simulink library "Garbage"  (R2023a)
settings: Solver=ode45  StartTime=0.0  StopTime=10.0  RelTol=1e-3  EnableMultiTasking=on
undefined symbols (not defined in the file or in its folder): c, ending_column_index, ending_row_index, framebuffer_16x1, framebuffer_2x2, framebuffer_4x4, framebuffer_8x8, HEIGHT, hot, POS_X, POS_Y, starting_column_index, starting_row_index, WIDTH
libraries: Memory_Blocks_Lib x459, simulink x235, simulink_extras x132, dspmtrx3 x116, Bit_Multiplexers_Lib x67, Bit_Demultiplexers_Lib x49, Byte_Multiplexers_Lib x40, Miscellaneous x38, Computer_Library x17, Decoders_Lib x14, commutil2 x12, Arithmetic_Operations_Lib x8
(part 11 of 26)

== system Garbage/NOT_USED_Byte_Demultiplexer_1_to_4 ==
  Addr_1(1b) : Inport
  Addr_0(1b) : Inport  Port=2
  Data(1B) : Inport  Port=3
  1_to_4_Bit_Demultiplexer(Bit_0) : Bit_Demultiplexers_Lib/Bit_Demultiplexers_Lib/Bit_Demultiplexer_1_to_4
  1_to_4_Bit_Demultiplexer(Bit_1) : Bit_Demultiplexers_Lib/Bit_Demultiplexers_Lib/Bit_Demultiplexer_1_to_4
  1_to_4_Bit_Demultiplexer(Bit_2) : Bit_Demultiplexers_Lib/Bit_Demultiplexers_Lib/Bit_Demultiplexer_1_to_4
  1_to_4_Bit_Demultiplexer(Bit_3) : Bit_Demultiplexers_Lib/Bit_Demultiplexers_Lib/Bit_Demultiplexer_1_to_4
  1_to_4_Bit_Demultiplexer(Bit_4) : Bit_Demultiplexers_Lib/Bit_Demultiplexers_Lib/Bit_Demultiplexer_1_to_4
  1_to_4_Bit_Demultiplexer(Bit_5) : Bit_Demultiplexers_Lib/Bit_Demultiplexers_Lib/Bit_Demultiplexer_1_to_4
  1_to_4_Bit_Demultiplexer(Bit_6) : Bit_Demultiplexers_Lib/Bit_Demultiplexers_Lib/Bit_Demultiplexer_1_to_4
  1_to_4_Bit_Demultiplexer(Bit_7) : Bit_Demultiplexers_Lib/Bit_Demultiplexers_Lib/Bit_Demultiplexer_1_to_4
  Bit to Integer Converter{,1..3} (4 alike) : commutil2/Bit to Integer Converter  nbits=8  bitOrder=MSB first  signedOutputValues=Unsigned  outDtype=uint8  outDtypeSigned=Inherit via internal rule
  Byte_{0..3} (4 alike) : Mux  Inputs=8
  Demux1 : Demux  Outputs=8
  Integer to Bit Converter7 : commutil2/Integer to Bit Converter  nbits=8  signedInputValues=Unsigned  bitOrder=MSB first  outDtype=boolean
  Data_3(1B) : Outport
  Data_2(1B) : Outport  Port=2
  Data_1(1B) : Outport  Port=3
  Data_0(1B) : Outport  Port=4
  wires:
  Bit to Integer Converter/1 -> Data_3(1B)/1
  Demux1/4 -> 1_to_4_Bit_Demultiplexer(Bit_4)/3
  Demux1/5 -> 1_to_4_Bit_Demultiplexer(Bit_3)/3
  Demux1/6 -> 1_to_4_Bit_Demultiplexer(Bit_2)/3
  Demux1/7 -> 1_to_4_Bit_Demultiplexer(Bit_1)/3
  Demux1/8 -> 1_to_4_Bit_Demultiplexer(Bit_0)/3
  Demux1/3 -> 1_to_4_Bit_Demultiplexer(Bit_5)/3
  Demux1/2 -> 1_to_4_Bit_Demultiplexer(Bit_6)/3
  Demux1/1 -> 1_to_4_Bit_Demultiplexer(Bit_7)/3
  Byte_3/1 -> Bit to Integer Converter/1
  Byte_2/1 -> Bit to Integer Converter1/1
  Byte_1/1 -> Bit to Integer Converter2/1
  Byte_0/1 -> Bit to Integer Converter3/1
  1_to_4_Bit_Demultiplexer(Bit_0)/4 -> Byte_0/8
  1_to_4_Bit_Demultiplexer(Bit_0)/3 -> Byte_1/8
  1_to_4_Bit_Demultiplexer(Bit_0)/2 -> Byte_2/8
  1_to_4_Bit_Demultiplexer(Bit_0)/1 -> Byte_3/8
  1_to_4_Bit_Demultiplexer(Bit_1)/4 -> Byte_0/7
  1_to_4_Bit_Demultiplexer(Bit_1)/3 -> Byte_1/7
  1_to_4_Bit_Demultiplexer(Bit_1)/2 -> Byte_2/7
  1_to_4_Bit_Demultiplexer(Bit_1)/1 -> Byte_3/7
  1_to_4_Bit_Demultiplexer(Bit_2)/4 -> Byte_0/6
  1_to_4_Bit_Demultiplexer(Bit_2)/3 -> Byte_1/6
  1_to_4_Bit_Demultiplexer(Bit_2)/2 -> Byte_2/6
  1_to_4_Bit_Demultiplexer(Bit_2)/1 -> Byte_3/6
  1_to_4_Bit_Demultiplexer(Bit_3)/4 -> Byte_0/5
  1_to_4_Bit_Demultiplexer(Bit_3)/3 -> Byte_1/5
  1_to_4_Bit_Demultiplexer(Bit_3)/2 -> Byte_2/5
  1_to_4_Bit_Demultiplexer(Bit_3)/1 -> Byte_3/5
  1_to_4_Bit_Demultiplexer(Bit_4)/4 -> Byte_0/4
  1_to_4_Bit_Demultiplexer(Bit_4)/3 -> Byte_1/4
  1_to_4_Bit_Demultiplexer(Bit_4)/2 -> Byte_2/4
  1_to_4_Bit_Demultiplexer(Bit_4)/1 -> Byte_3/4
  1_to_4_Bit_Demultiplexer(Bit_5)/4 -> Byte_0/3
  1_to_4_Bit_Demultiplexer(Bit_5)/3 -> Byte_1/3
  1_to_4_Bit_Demultiplexer(Bit_5)/2 -> Byte_2/3
  1_to_4_Bit_Demultiplexer(Bit_5)/1 -> Byte_3/3
  1_to_4_Bit_Demultiplexer(Bit_6)/4 -> Byte_0/2
  1_to_4_Bit_Demultiplexer(Bit_6)/3 -> Byte_1/2
  1_to_4_Bit_Demultiplexer(Bit_6)/2 -> Byte_2/2
  1_to_4_Bit_Demultiplexer(Bit_6)/1 -> Byte_3/2
  1_to_4_Bit_Demultiplexer(Bit_7)/4 -> Byte_0/1
  1_to_4_Bit_Demultiplexer(Bit_7)/3 -> Byte_1/1
  1_to_4_Bit_Demultiplexer(Bit_7)/2 -> Byte_2/1
  1_to_4_Bit_Demultiplexer(Bit_7)/1 -> Byte_3/1
  Integer to Bit Converter7/1 -> Demux1/1
  Data(1B)/1 -> Integer to Bit Converter7/1
  Addr_0(1b)/1 -> 1_to_4_Bit_Demultiplexer(Bit_7)/2, 1_to_4_Bit_Demultiplexer(Bit_3)/2, 1_to_4_Bit_Demultiplexer(Bit_2)/2, 1_to_4_Bit_Demultiplexer(Bit_1)/2, 1_to_4_Bit_Demultiplexer(Bit_0)/2, 1_to_4_Bit_Demultiplexer(Bit_4)/2, 1_to_4_Bit_Demultiplexer(Bit_5)/2, 1_to_4_Bit_Demultiplexer(Bit_6)/2
  Addr_1(1b)/1 -> 1_to_4_Bit_Demultiplexer(Bit_7)/1, 1_to_4_Bit_Demultiplexer(Bit_0)/1, 1_to_4_Bit_Demultiplexer(Bit_1)/1, 1_to_4_Bit_Demultiplexer(Bit_2)/1, 1_to_4_Bit_Demultiplexer(Bit_3)/1, 1_to_4_Bit_Demultiplexer(Bit_4)/1, 1_to_4_Bit_Demultiplexer(Bit_5)/1, 1_to_4_Bit_Demultiplexer(Bit_6)/1
  Bit to Integer Converter1/1 -> Data_2(1B)/1
  Bit to Integer Converter2/1 -> Data_1(1B)/1
  Bit to Integer Converter3/1 -> Data_0(1B)/1

== system Garbage/NOT_USED_Byte_Demultiplexer_1_to_8 ==
  Addr_2(1b) : Inport
  Addr_1(1b) : Inport  Port=2
  Addr_0(1b) : Inport  Port=3
  Data(1B) : Inport  Port=4
  1_to_8_Bit_Demultiplexer(Bit_0) : Bit_Demultiplexers_Lib/Bit_Demultiplexers_Lib/Bit_Demultiplexer_1_to_8
  1_to_8_Bit_Demultiplexer(Bit_1) : Bit_Demultiplexers_Lib/Bit_Demultiplexers_Lib/Bit_Demultiplexer_1_to_8
  1_to_8_Bit_Demultiplexer(Bit_2) : Bit_Demultiplexers_Lib/Bit_Demultiplexers_Lib/Bit_Demultiplexer_1_to_8
  1_to_8_Bit_Demultiplexer(Bit_3) : Bit_Demultiplexers_Lib/Bit_Demultiplexers_Lib/Bit_Demultiplexer_1_to_8
  1_to_8_Bit_Demultiplexer(Bit_4) : Bit_Demultiplexers_Lib/Bit_Demultiplexers_Lib/Bit_Demultiplexer_1_to_8
  1_to_8_Bit_Demultiplexer(Bit_5) : Bit_Demultiplexers_Lib/Bit_Demultiplexers_Lib/Bit_Demultiplexer_1_to_8
  1_to_8_Bit_Demultiplexer(Bit_6) : Bit_Demultiplexers_Lib/Bit_Demultiplexers_Lib/Bit_Demultiplexer_1_to_8
  1_to_8_Bit_Demultiplexer(Bit_7) : Bit_Demultiplexers_Lib/Bit_Demultiplexers_Lib/Bit_Demultiplexer_1_to_8
  Bus Creator{1..7,15} (8 alike) : BusCreator  Inputs=8
  Demux1 : Demux  Outputs=8
  Data_7(1B) : Outport
  Data_6(1B) : Outport  Port=2
  Data_5(1B) : Outport  Port=3
  Data_4(1B) : Outport  Port=4
  Data_3(1B) : Outport  Port=5
  Data_2(1B) : Outport  Port=6
  Data_1(1B) : Outport  Port=7
  Data_0(1B) : Outport  Port=8
  wires:
  Demux1/4 -> 1_to_8_Bit_Demultiplexer(Bit_4)/4
  Demux1/5 -> 1_to_8_Bit_Demultiplexer(Bit_3)/4
  Demux1/6 -> 1_to_8_Bit_Demultiplexer(Bit_2)/4
  Demux1/7 -> 1_to_8_Bit_Demultiplexer(Bit_1)/4
  Demux1/8 -> 1_to_8_Bit_Demultiplexer(Bit_0)/4
  Demux1/3 -> 1_to_8_Bit_Demultiplexer(Bit_5)/4
  Demux1/2 -> 1_to_8_Bit_Demultiplexer(Bit_6)/4
  Demux1/1 -> 1_to_8_Bit_Demultiplexer(Bit_7)/4
  1_to_8_Bit_Demultiplexer(Bit_0)/8 -> Bus Creator7/8
  1_to_8_Bit_Demultiplexer(Bit_0)/7 -> Bus Creator6/8
  1_to_8_Bit_Demultiplexer(Bit_0)/6 -> Bus Creator5/8
  1_to_8_Bit_Demultiplexer(Bit_0)/5 -> Bus Creator4/8
  1_to_8_Bit_Demultiplexer(Bit_0)/4 -> Bus Creator3/8
  1_to_8_Bit_Demultiplexer(Bit_0)/3 -> Bus Creator2/8
  1_to_8_Bit_Demultiplexer(Bit_0)/2 -> Bus Creator1/8
  1_to_8_Bit_Demultiplexer(Bit_0)/1 -> Bus Creator15/8
  1_to_8_Bit_Demultiplexer(Bit_1)/8 -> Bus Creator7/7
  1_to_8_Bit_Demultiplexer(Bit_1)/7 -> Bus Creator6/7
  1_to_8_Bit_Demultiplexer(Bit_1)/6 -> Bus Creator5/7
  1_to_8_Bit_Demultiplexer(Bit_1)/5 -> Bus Creator4/7
  1_to_8_Bit_Demultiplexer(Bit_1)/4 -> Bus Creator3/7
  1_to_8_Bit_Demultiplexer(Bit_1)/3 -> Bus Creator2/7
  1_to_8_Bit_Demultiplexer(Bit_1)/2 -> Bus Creator1/7
  1_to_8_Bit_Demultiplexer(Bit_1)/1 -> Bus Creator15/7
  1_to_8_Bit_Demultiplexer(Bit_2)/8 -> Bus Creator7/6
  1_to_8_Bit_Demultiplexer(Bit_2)/7 -> Bus Creator6/6
  1_to_8_Bit_Demultiplexer(Bit_2)/6 -> Bus Creator5/6
  1_to_8_Bit_Demultiplexer(Bit_2)/5 -> Bus Creator4/6
  1_to_8_Bit_Demultiplexer(Bit_2)/4 -> Bus Creator3/6
  1_to_8_Bit_Demultiplexer(Bit_2)/3 -> Bus Creator2/6
  1_to_8_Bit_Demultiplexer(Bit_2)/2 -> Bus Creator1/6
  1_to_8_Bit_Demultiplexer(Bit_2)/1 -> Bus Creator15/6
  1_to_8_Bit_Demultiplexer(Bit_3)/8 -> Bus Creator7/5
  1_to_8_Bit_Demultiplexer(Bit_3)/7 -> Bus Creator6/5
  1_to_8_Bit_Demultiplexer(Bit_3)/6 -> Bus Creator5/5
  1_to_8_Bit_Demultiplexer(Bit_3)/5 -> Bus Creator4/5
  1_to_8_Bit_Demultiplexer(Bit_3)/4 -> Bus Creator3/5
  1_to_8_Bit_Demultiplexer(Bit_3)/3 -> Bus Creator2/5
  1_to_8_Bit_Demultiplexer(Bit_3)/2 -> Bus Creator1/5
  1_to_8_Bit_Demultiplexer(Bit_3)/1 -> Bus Creator15/5
  1_to_8_Bit_Demultiplexer(Bit_4)/8 -> Bus Creator7/4
  1_to_8_Bit_Demultiplexer(Bit_4)/7 -> Bus Creator6/4
  1_to_8_Bit_Demultiplexer(Bit_4)/6 -> Bus Creator5/4
  1_to_8_Bit_Demultiplexer(Bit_4)/5 -> Bus Creator4/4
  1_to_8_Bit_Demultiplexer(Bit_4)/4 -> Bus Creator3/4
  1_to_8_Bit_Demultiplexer(Bit_4)/3 -> Bus Creator2/4
  1_to_8_Bit_Demultiplexer(Bit_4)/2 -> Bus Creator1/4
  1_to_8_Bit_Demultiplexer(Bit_4)/1 -> Bus Creator15/4
  1_to_8_Bit_Demultiplexer(Bit_5)/8 -> Bus Creator7/3
  1_to_8_Bit_Demultiplexer(Bit_5)/7 -> Bus Creator6/3
  1_to_8_Bit_Demultiplexer(Bit_5)/6 -> Bus Creator5/3
  1_to_8_Bit_Demultiplexer(Bit_5)/5 -> Bus Creator4/3
  1_to_8_Bit_Demultiplexer(Bit_5)/4 -> Bus Creator3/3
  1_to_8_Bit_Demultiplexer(Bit_5)/3 -> Bus Creator2/3
  1_to_8_Bit_Demultiplexer(Bit_5)/2 -> Bus Creator1/3
  1_to_8_Bit_Demultiplexer(Bit_5)/1 -> Bus Creator15/3
  1_to_8_Bit_Demultiplexer(Bit_6)/8 -> Bus Creator7/2
  1_to_8_Bit_Demultiplexer(Bit_6)/7 -> Bus Creator6/2
  1_to_8_Bit_Demultiplexer(Bit_6)/6 -> Bus Creator5/2
  1_to_8_Bit_Demultiplexer(Bit_6)/5 -> Bus Creator4/2
  1_to_8_Bit_Demultiplexer(Bit_6)/4 -> Bus Creator3/2
  1_to_8_Bit_Demultiplexer(Bit_6)/3 -> Bus Creator2/2
  1_to_8_Bit_Demultiplexer(Bit_6)/2 -> Bus Creator1/2
  1_to_8_Bit_Demultiplexer(Bit_6)/1 -> Bus Creator15/2
  1_to_8_Bit_Demultiplexer(Bit_7)/8 -> Bus Creator7/1
  1_to_8_Bit_Demultiplexer(Bit_7)/7 -> Bus Creator6/1
  1_to_8_Bit_Demultiplexer(Bit_7)/6 -> Bus Creator5/1
  1_to_8_Bit_Demultiplexer(Bit_7)/5 -> Bus Creator4/1
  1_to_8_Bit_Demultiplexer(Bit_7)/4 -> Bus Creator3/1
  1_to_8_Bit_Demultiplexer(Bit_7)/3 -> Bus Creator2/1
  1_to_8_Bit_Demultiplexer(Bit_7)/2 -> Bus Creator1/1
  1_to_8_Bit_Demultiplexer(Bit_7)/1 -> Bus Creator15/1
  Data(1B)/1 -> Demux1/1
  Addr_0(1b)/1 -> 1_to_8_Bit_Demultiplexer(Bit_7)/3, 1_to_8_Bit_Demultiplexer(Bit_6)/3, 1_to_8_Bit_Demultiplexer(Bit_5)/3, 1_to_8_Bit_Demultiplexer(Bit_0)/3, 1_to_8_Bit_Demultiplexer(Bit_1)/3, 1_to_8_Bit_Demultiplexer(Bit_2)/3, 1_to_8_Bit_Demultiplexer(Bit_3)/3, 1_to_8_Bit_Demultiplexer(Bit_4)/3
  Addr_1(1b)/1 -> 1_to_8_Bit_Demultiplexer(Bit_7)/2, 1_to_8_Bit_Demultiplexer(Bit_0)/2, 1_to_8_Bit_Demultiplexer(Bit_1)/2, 1_to_8_Bit_Demultiplexer(Bit_2)/2, 1_to_8_Bit_Demultiplexer(Bit_3)/2, 1_to_8_Bit_Demultiplexer(Bit_4)/2, 1_to_8_Bit_Demultiplexer(Bit_5)/2, 1_to_8_Bit_Demultiplexer(Bit_6)/2
  Addr_2(1b)/1 -> 1_to_8_Bit_Demultiplexer(Bit_7)/1, 1_to_8_Bit_Demultiplexer(Bit_0)/1, 1_to_8_Bit_Demultiplexer(Bit_1)/1, 1_to_8_Bit_Demultiplexer(Bit_2)/1, 1_to_8_Bit_Demultiplexer(Bit_3)/1, 1_to_8_Bit_Demultiplexer(Bit_4)/1, 1_to_8_Bit_Demultiplexer(Bit_5)/1, 1_to_8_Bit_Demultiplexer(Bit_6)/1
  Bus Creator7/1 -> Data_0(1B)/1
  Bus Creator6/1 -> Data_1(1B)/1
  Bus Creator5/1 -> Data_2(1B)/1
  Bus Creator4/1 -> Data_3(1B)/1
  Bus Creator3/1 -> Data_4(1B)/1
  Bus Creator2/1 -> Data_5(1B)/1
  Bus Creator1/1 -> Data_6(1B)/1
  Bus Creator15/1 -> Data_7(1B)/1

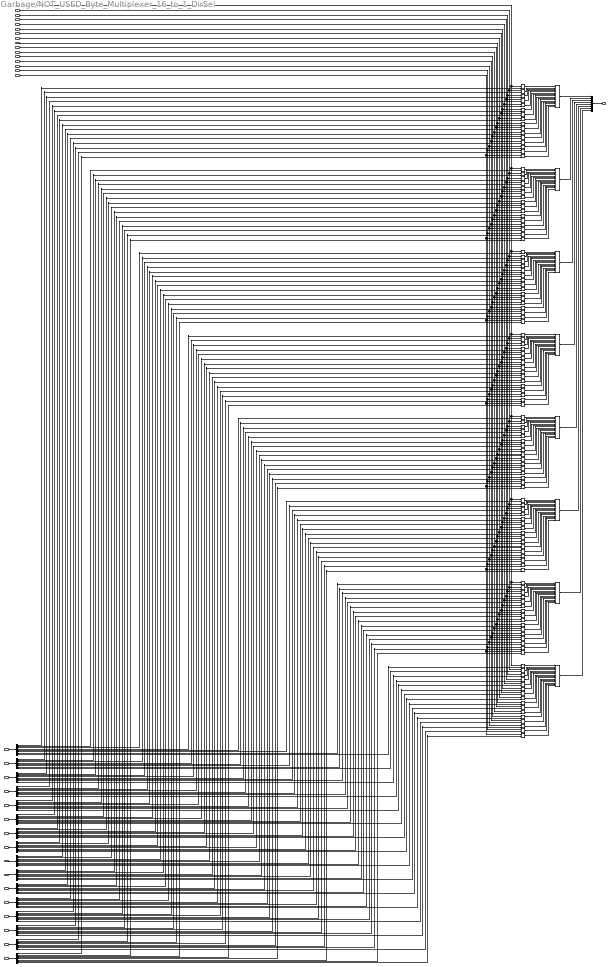
== system Garbage/NOT_USED_Byte_Multiplexer_16_to_1_DirSel ==
  note: Bit_Multiplexer_16_to_1_DirSel(Bit_07)
  note: Bit_Multiplexer_16_to_1_DirSel(Bit_06)
  note: Bit_Multiplexer_16_to_1_DirSel(Bit_05)
  note: Bit_Multiplexer_16_to_1_DirSel(Bit_04)
  note: Bit_Multiplexer_16_to_1_DirSel(Bit_03)
  note: Bit_Multiplexer_16_to_1_DirSel(Bit_02)
  note: Bit_Multiplexer_16_to_1_DirSel(Bit_01)
  note: Bit_Multiplexer_16_to_1_DirSel(Bit_00)
  Select_15(1b) : Inport
  Select_14(1b) : Inport  Port=2
  Select_13(1b) : Inport  Port=3
  Select_12(1b) : Inport  Port=4
  Select_11(1b) : Inport  Port=5
  Select_10(1b) : Inport  Port=6
  Select_09(1b) : Inport  Port=7
  Select_08(1b) : Inport  Port=8
  Select_07(1b) : Inport  Port=9
  Select_06(1b) : Inport  Port=10
  Select_05(1b) : Inport  Port=11
  Select_04(1b) : Inport  Port=12
  Select_03(1b) : Inport  Port=13
  Select_02(1b) : Inport  Port=14
  Select_01(1b) : Inport  Port=15
  Select_00(1b) : Inport  Port=16
  Data_15(1B) : Inport  Port=17
  Data_14(1B) : Inport  Port=18
  Data_13(1B) : Inport  Port=19
  Data_12(1B) : Inport  Port=20
  Data_11(1B) : Inport  Port=21
  Data_10(1B) : Inport  Port=22
  Data_09(1B) : Inport  Port=23
  Data_08(1B) : Inport  Port=24
  Data_07(1B) : Inport  Port=25
  Data_06(1B) : Inport  Port=26
  Data_05(1B) : Inport  Port=27
  Data_04(1B) : Inport  Port=28
  Data_03(1B) : Inport  Port=29
  Data_02(1B) : Inport  Port=30
  Data_01(1B) : Inport  Port=31
  Data_00(1B) : Inport  Port=32
  Bus Creator : BusCreator  Inputs=8
  Bus Selector{1..16} (16 alike) : BusSelector  OutputSignals=signal1,signal2,signal3,signal4,signal5,signal6,signal7,signal8
  Logical Operator{137..151,153..168,170..185,187..202,204..219,221..236,238..253,255..270,272} (128 alike) : Logic  IconShape=distinctive  AllPortsSameDT=off  OutDataTypeStr=boolean
  Logical Operator{152,169,186,203,220,237,254,271} (8 alike) : Logic  Operator=OR  Inputs=16  IconShape=distinctive  OutDataTypeStr=boolean
  Data(1B) : Outport
  wires:
  Data_15(1B)/1 -> Bus Selector15/1
  Data_14(1B)/1 -> Bus Selector1/1
  Data_13(1B)/1 -> Bus Selector2/1
  Data_12(1B)/1 -> Bus Selector3/1
  Data_11(1B)/1 -> Bus Selector4/1
  Data_10(1B)/1 -> Bus Selector5/1
  Data_09(1B)/1 -> Bus Selector6/1
  Data_08(1B)/1 -> Bus Selector7/1
  Data_07(1B)/1 -> Bus Selector8/1
  Data_06(1B)/1 -> Bus Selector9/1
  Data_05(1B)/1 -> Bus Selector10/1
  Data_04(1B)/1 -> Bus Selector11/1
  Data_03(1B)/1 -> Bus Selector12/1
  Data_02(1B)/1 -> Bus Selector13/1
  Data_01(1B)/1 -> Bus Selector14/1
  Data_00(1B)/1 -> Bus Selector16/1
  Bus Creator/1 -> Data(1B)/1
  Bus Selector16/8 -> Logical Operator264/2  "<signal8>"
  Bus Selector14/8 -> Logical Operator263/2  "<signal8>"
  Bus Selector13/8 -> Logical Operator262/2  "<signal8>"
  Bus Selector12/8 -> Logical Operator261/2  "<signal8>"
  Bus Selector11/8 -> Logical Operator258/2  "<signal8>"
  Bus Selector10/8 -> Logical Operator257/2  "<signal8>"
  Bus Selector9/8 -> Logical Operator256/2  "<signal8>"
  Bus Selector8/8 -> Logical Operator272/2  "<signal8>"
  Bus Selector15/8 -> Logical Operator259/2  "<signal8>"
  Bus Selector1/8 -> Logical Operator260/2  "<signal8>"
  Bus Selector2/8 -> Logical Operator265/2  "<signal8>"
  Bus Selector3/8 -> Logical Operator266/2  "<signal8>"
  Bus Selector4/8 -> Logical Operator267/2  "<signal8>"
  Bus Selector5/8 -> Logical Operator268/2  "<signal8>"
  Bus Selector6/8 -> Logical Operator269/2  "<signal8>"
  Bus Selector7/8 -> Logical Operator270/2  "<signal8>"
  Bus Selector16/7 -> Logical Operator247/2  "<signal7>"
  Bus Selector14/7 -> Logical Operator246/2  "<signal7>"
  Bus Selector13/7 -> Logical Operator245/2  "<signal7>"
  Bus Selector12/7 -> Logical Operator244/2  "<signal7>"
  Bus Selector11/7 -> Logical Operator241/2  "<signal7>"
  Bus Selector10/7 -> Logical Operator240/2  "<signal7>"
  Bus Selector9/7 -> Logical Operator239/2  "<signal7>"
  Bus Selector8/7 -> Logical Operator255/2  "<signal7>"
  Bus Selector15/7 -> Logical Operator242/2  "<signal7>"
  Bus Selector1/7 -> Logical Operator243/2  "<signal7>"
  Bus Selector2/7 -> Logical Operator248/2  "<signal7>"
  Bus Selector3/7 -> Logical Operator249/2  "<signal7>"
  Bus Selector4/7 -> Logical Operator250/2  "<signal7>"
  Bus Selector5/7 -> Logical Operator251/2  "<signal7>"
  Bus Selector6/7 -> Logical Operator252/2  "<signal7>"
  Bus Selector7/7 -> Logical Operator253/2  "<signal7>"
  Bus Selector16/6 -> Logical Operator230/2  "<signal6>"
  Bus Selector14/6 -> Logical Operator229/2  "<signal6>"
  Bus Selector13/6 -> Logical Operator228/2  "<signal6>"
  Bus Selector12/6 -> Logical Operator227/2  "<signal6>"
  Bus Selector11/6 -> Logical Operator224/2  "<signal6>"
  Bus Selector10/6 -> Logical Operator223/2  "<signal6>"
  Bus Selector9/6 -> Logical Operator222/2  "<signal6>"
  Bus Selector8/6 -> Logical Operator238/2  "<signal6>"
  Bus Selector15/6 -> Logical Operator225/2  "<signal6>"
  Bus Selector1/6 -> Logical Operator226/2  "<signal6>"
  Bus Selector2/6 -> Logical Operator231/2  "<signal6>"
  Bus Selector3/6 -> Logical Operator232/2  "<signal6>"
  Bus Selector4/6 -> Logical Operator233/2  "<signal6>"
  Bus Selector5/6 -> Logical Operator234/2  "<signal6>"
  Bus Selector6/6 -> Logical Operator235/2  "<signal6>"
  Bus Selector7/6 -> Logical Operator236/2  "<signal6>"
  Bus Selector16/5 -> Logical Operator213/2  "<signal5>"
  Bus Selector14/5 -> Logical Operator212/2  "<signal5>"
  Bus Selector13/5 -> Logical Operator211/2  "<signal5>"
  Bus Selector12/5 -> Logical Operator210/2  "<signal5>"
  Bus Selector11/5 -> Logical Operator207/2  "<signal5>"
  Bus Selector10/5 -> Logical Operator206/2  "<signal5>"
  Bus Selector9/5 -> Logical Operator205/2  "<signal5>"
  Bus Selector8/5 -> Logical Operator221/2  "<signal5>"
  Bus Selector15/5 -> Logical Operator208/2  "<signal5>"
  Bus Selector1/5 -> Logical Operator209/2  "<signal5>"
  Bus Selector2/5 -> Logical Operator214/2  "<signal5>"
  Bus Selector3/5 -> Logical Operator215/2  "<signal5>"
  Bus Selector4/5 -> Logical Operator216/2  "<signal5>"
  Bus Selector5/5 -> Logical Operator217/2  "<signal5>"
  Bus Selector6/5 -> Logical Operator218/2  "<signal5>"
  Bus Selector7/5 -> Logical Operator219/2  "<signal5>"
  Bus Selector16/4 -> Logical Operator196/2  "<signal4>"
  Bus Selector14/4 -> Logical Operator195/2  "<signal4>"
  Bus Selector13/4 -> Logical Operator194/2  "<signal4>"
  Bus Selector12/4 -> Logical Operator193/2  "<signal4>"
  Bus Selector11/4 -> Logical Operator190/2  "<signal4>"
  Bus Selector10/4 -> Logical Operator189/2  "<signal4>"
  Bus Selector9/4 -> Logical Operator188/2  "<signal4>"
  Bus Selector8/4 -> Logical Operator204/2  "<signal4>"
  Bus Selector15/4 -> Logical Operator191/2  "<signal4>"
  Bus Selector1/4 -> Logical Operator192/2  "<signal4>"
  Bus Selector2/4 -> Logical Operator197/2  "<signal4>"
  Bus Selector3/4 -> Logical Operator198/2  "<signal4>"
  Bus Selector4/4 -> Logical Operator199/2  "<signal4>"
  Bus Selector5/4 -> Logical Operator200/2  "<signal4>"
  Bus Selector6/4 -> Logical Operator201/2  "<signal4>"
  Bus Selector7/4 -> Logical Operator202/2  "<signal4>"
  Bus Selector16/3 -> Logical Operator179/2  "<signal3>"
  Bus Selector14/3 -> Logical Operator178/2  "<signal3>"
  Bus Selector13/3 -> Logical Operator177/2  "<signal3>"
  Bus Selector12/3 -> Logical Operator176/2  "<signal3>"
  Bus Selector11/3 -> Logical Operator173/2  "<signal3>"
  Bus Selector10/3 -> Logical Operator172/2  "<signal3>"
  Bus Selector9/3 -> Logical Operator171/2  "<signal3>"
  Bus Selector8/3 -> Logical Operator187/2  "<signal3>"
  Bus Selector15/3 -> Logical Operator174/2  "<signal3>"
  Bus Selector1/3 -> Logical Operator175/2  "<signal3>"
  Bus Selector2/3 -> Logical Operator180/2  "<signal3>"
  Bus Selector3/3 -> Logical Operator181/2  "<signal3>"
  Bus Selector4/3 -> Logical Operator182/2  "<signal3>"
  Bus Selector5/3 -> Logical Operator183/2  "<signal3>"
  Bus Selector6/3 -> Logical Operator184/2  "<signal3>"
  Bus Selector7/3 -> Logical Operator185/2  "<signal3>"
  Bus Selector16/2 -> Logical Operator162/2  "<signal2>"
  Bus Selector14/2 -> Logical Operator161/2  "<signal2>"
  Bus Selector13/2 -> Logical Operator160/2  "<signal2>"
  Bus Selector12/2 -> Logical Operator159/2  "<signal2>"
  Bus Selector11/2 -> Logical Operator156/2  "<signal2>"
  Bus Selector10/2 -> Logical Operator155/2  "<signal2>"
  Bus Selector9/2 -> Logical Operator154/2  "<signal2>"
  Bus Selector8/2 -> Logical Operator170/2  "<signal2>"
  Bus Selector15/2 -> Logical Operator157/2  "<signal2>"
  Bus Selector1/2 -> Logical Operator158/2  "<signal2>"
  Bus Selector2/2 -> Logical Operator163/2  "<signal2>"
  Bus Selector3/2 -> Logical Operator164/2  "<signal2>"
  Bus Selector4/2 -> Logical Operator165/2  "<signal2>"
  Bus Selector5/2 -> Logical Operator166/2  "<signal2>"
  Bus Selector6/2 -> Logical Operator167/2  "<signal2>"
  Bus Selector7/2 -> Logical Operator168/2  "<signal2>"
  Bus Selector16/1 -> Logical Operator145/2  "<signal1>"
  Bus Selector14/1 -> Logical Operator144/2  "<signal1>"
  Bus Selector13/1 -> Logical Operator143/2  "<signal1>"
  Bus Selector12/1 -> Logical Operator142/2  "<signal1>"
  Bus Selector11/1 -> Logical Operator139/2  "<signal1>"
  Bus Selector10/1 -> Logical Operator138/2  "<signal1>"
  Bus Selector9/1 -> Logical Operator137/2  "<signal1>"
  Bus Selector8/1 -> Logical Operator153/2  "<signal1>"
  Bus Selector15/1 -> Logical Operator140/2  "<signal1>"
  Bus Selector1/1 -> Logical Operator141/2  "<signal1>"
  Bus Selector2/1 -> Logical Operator146/2  "<signal1>"
  Bus Selector3/1 -> Logical Operator147/2  "<signal1>"
  Bus Selector4/1 -> Logical Operator148/2  "<signal1>"
  Bus Selector5/1 -> Logical Operator149/2  "<signal1>"
  Bus Selector6/1 -> Logical Operator150/2  "<signal1>"
  Bus Selector7/1 -> Logical Operator151/2  "<signal1>"
  Select_15(1b)/1 -> Logical Operator225/1, Logical Operator259/1, Logical Operator242/1, Logical Operator208/1, Logical Operator191/1, Logical Operator174/1, Logical Operator157/1, Logical Operator140/1
  Select_14(1b)/1 -> Logical Operator260/1, Logical Operator243/1, Logical Operator226/1, Logical Operator209/1, Logical Operator192/1, Logical Operator175/1, Logical Operator158/1, Logical Operator141/1
  Select_13(1b)/1 -> Logical Operator265/1, Logical Operator248/1, Logical Operator231/1, Logical Operator214/1, Logical Operator197/1, Logical Operator180/1, Logical Operator163/1, Logical Operator146/1
  Select_12(1b)/1 -> Logical Operator266/1, Logical Operator249/1, Logical Operator232/1, Logical Operator215/1, Logical Operator198/1, Logical Operator181/1, Logical Operator164/1, Logical Operator147/1
  Select_11(1b)/1 -> Logical Operator267/1, Logical Operator250/1, Logical Operator233/1, Logical Operator216/1, Logical Operator199/1, Logical Operator182/1, Logical Operator165/1, Logical Operator148/1
  Select_10(1b)/1 -> Logical Operator268/1, Logical Operator251/1, Logical Operator234/1, Logical Operator217/1, Logical Operator200/1, Logical Operator183/1, Logical Operator166/1, Logical Operator149/1
  Select_09(1b)/1 -> Logical Operator269/1, Logical Operator252/1, Logical Operator235/1, Logical Operator218/1, Logical Operator201/1, Logical Operator184/1, Logical Operator167/1, Logical Operator150/1
  Select_08(1b)/1 -> Logical Operator270/1, Logical Operator253/1, Logical Operator236/1, Logical Operator219/1, Logical Operator202/1, Logical Operator185/1, Logical Operator168/1, Logical Operator151/1
  Select_07(1b)/1 -> Logical Operator272/1, Logical Operator255/1, Logical Operator238/1, Logical Operator221/1, Logical Operator204/1, Logical Operator187/1, Logical Operator170/1, Logical Operator153/1
  Select_06(1b)/1 -> Logical Operator256/1, Logical Operator239/1, Logical Operator222/1, Logical Operator205/1, Logical Operator188/1, Logical Operator171/1, Logical Operator154/1, Logical Operator137/1
  Select_04(1b)/1 -> Logical Operator258/1, Logical Operator241/1, Logical Operator224/1, Logical Operator207/1, Logical Operator190/1, Logical Operator173/1, Logical Operator156/1, Logical Operator139/1
  Select_03(1b)/1 -> Logical Operator261/1, Logical Operator244/1, Logical Operator227/1, Logical Operator210/1, Logical Operator193/1, Logical Operator176/1, Logical Operator159/1, Logical Operator142/1
  Select_02(1b)/1 -> Logical Operator262/1, Logical Operator245/1, Logical Operator228/1, Logical Operator211/1, Logical Operator194/1, Logical Operator177/1, Logical Operator160/1, Logical Operator143/1
  Select_00(1b)/1 -> Logical Operator264/1, Logical Operator247/1, Logical Operator230/1, Logical Operator213/1, Logical Operator196/1, Logical Operator179/1, Logical Operator162/1, Logical Operator145/1
  Logical Operator147/1 -> Logical Operator152/4
  Logical Operator146/1 -> Logical Operator152/3
  Logical Operator141/1 -> Logical Operator152/2
  Logical Operator140/1 -> Logical Operator152/1
  Logical Operator151/1 -> Logical Operator152/8
  Logical Operator150/1 -> Logical Operator152/7
  Logical Operator149/1 -> Logical Operator152/6
  Logical Operator148/1 -> Logical Operator152/5
  Logical Operator139/1 -> Logical Operator152/12
  Logical Operator138/1 -> Logical Operator152/11
  Logical Operator137/1 -> Logical Operator152/10
  Logical Operator153/1 -> Logical Operator152/9
  Logical Operator145/1 -> Logical Operator152/16
  Logical Operator144/1 -> Logical Operator152/15
  Logical Operator143/1 -> Logical Operator152/14
  Logical Operator142/1 -> Logical Operator152/13
  Logical Operator169/1 -> Bus Creator/2
  Logical Operator164/1 -> Logical Operator169/4
  Logical Operator163/1 -> Logical Operator169/3
  Logical Operator158/1 -> Logical Operator169/2
  Logical Operator157/1 -> Logical Operator169/1
  Logical Operator168/1 -> Logical Operator169/8
  Logical Operator167/1 -> Logical Operator169/7
  Logical Operator166/1 -> Logical Operator169/6
  Logical Operator165/1 -> Logical Operator169/5
  Logical Operator156/1 -> Logical Operator169/12
  Logical Operator155/1 -> Logical Operator169/11
  Logical Operator154/1 -> Logical Operator169/10
  Logical Operator170/1 -> Logical Operator169/9
  Logical Operator162/1 -> Logical Operator169/16
  Logical Operator161/1 -> Logical Operator169/15
  Logical Operator160/1 -> Logical Operator169/14
  Logical Operator159/1 -> Logical Operator169/13
  Logical Operator181/1 -> Logical Operator186/4
  Logical Operator180/1 -> Logical Operator186/3
  Logical Operator175/1 -> Logical Operator186/2
  Logical Operator174/1 -> Logical Operator186/1
  Logical Operator185/1 -> Logical Operator186/8
  Logical Operator184/1 -> Logical Operator186/7
  Logical Operator183/1 -> Logical Operator186/6
  Logical Operator182/1 -> Logical Operator186/5
  Logical Operator173/1 -> Logical Operator186/12
  Logical Operator172/1 -> Logical Operator186/11
  Logical Operator171/1 -> Logical Operator186/10
  Logical Operator187/1 -> Logical Operator186/9
  Logical Operator179/1 -> Logical Operator186/16
  Logical Operator178/1 -> Logical Operator186/15
  Logical Operator177/1 -> Logical Operator186/14
  Logical Operator176/1 -> Logical Operator186/13
  Logical Operator203/1 -> Bus Creator/4
  Logical Operator198/1 -> Logical Operator203/4
  Logical Operator197/1 -> Logical Operator203/3
  Logical Operator192/1 -> Logical Operator203/2
  Logical Operator191/1 -> Logical Operator203/1
  Logical Operator202/1 -> Logical Operator203/8
  Logical Operator201/1 -> Logical Operator203/7
  Logical Operator200/1 -> Logical Operator203/6
  Logical Operator199/1 -> Logical Operator203/5
  Logical Operator190/1 -> Logical Operator203/12
  Logical Operator189/1 -> Logical Operator203/11
  Logical Operator188/1 -> Logical Operator203/10
  Logical Operator204/1 -> Logical Operator203/9
  Logical Operator196/1 -> Logical Operator203/16
  Logical Operator195/1 -> Logical Operator203/15
  Logical Operator194/1 -> Logical Operator203/14
  Logical Operator193/1 -> Logical Operator203/13
  Logical Operator215/1 -> Logical Operator220/4
  Logical Operator214/1 -> Logical Operator220/3
  Logical Operator209/1 -> Logical Operator220/2
  Logical Operator208/1 -> Logical Operator220/1
  Logical Operator219/1 -> Logical Operator220/8
  Logical Operator218/1 -> Logical Operator220/7
  Logical Operator217/1 -> Logical Operator220/6
  Logical Operator216/1 -> Logical Operator220/5
  Logical Operator207/1 -> Logical Operator220/12
  Logical Operator206/1 -> Logical Operator220/11
  Logical Operator205/1 -> Logical Operator220/10
  Logical Operator221/1 -> Logical Operator220/9
  Logical Operator213/1 -> Logical Operator220/16
  Logical Operator212/1 -> Logical Operator220/15
  Logical Operator211/1 -> Logical Operator220/14
  Logical Operator210/1 -> Logical Operator220/13
  Logical Operator237/1 -> Bus Creator/6
  Logical Operator232/1 -> Logical Operator237/4
  Logical Operator231/1 -> Logical Operator237/3
  Logical Operator226/1 -> Logical Operator237/2
  Logical Operator225/1 -> Logical Operator237/1
  Logical Operator236/1 -> Logical Operator237/8
  Logical Operator235/1 -> Logical Operator237/7
  Logical Operator234/1 -> Logical Operator237/6
  Logical Operator233/1 -> Logical Operator237/5
  Logical Operator224/1 -> Logical Operator237/12
  Logical Operator223/1 -> Logical Operator237/11
  Logical Operator222/1 -> Logical Operator237/10
  Logical Operator238/1 -> Logical Operator237/9
  Logical Operator230/1 -> Logical Operator237/16
  Logical Operator229/1 -> Logical Operator237/15
  Logical Operator228/1 -> Logical Operator237/14
  Logical Operator227/1 -> Logical Operator237/13
  Logical Operator249/1 -> Logical Operator254/4
  Logical Operator248/1 -> Logical Operator254/3
  Logical Operator243/1 -> Logical Operator254/2
  Logical Operator242/1 -> Logical Operator254/1
  Logical Operator253/1 -> Logical Operator254/8
  Logical Operator252/1 -> Logical Operator254/7
  Logical Operator251/1 -> Logical Operator254/6
  Logical Operator250/1 -> Logical Operator254/5
  Logical Operator241/1 -> Logical Operator254/12
  Logical Operator240/1 -> Logical Operator254/11
  Logical Operator239/1 -> Logical Operator254/10
  Logical Operator255/1 -> Logical Operator254/9
  Logical Operator247/1 -> Logical Operator254/16
  Logical Operator246/1 -> Logical Operator254/15
  Logical Operator245/1 -> Logical Operator254/14
  Logical Operator244/1 -> Logical Operator254/13
  Logical Operator271/1 -> Bus Creator/8
  Logical Operator266/1 -> Logical Operator271/4
  Logical Operator265/1 -> Logical Operator271/3
  Logical Operator260/1 -> Logical Operator271/2
  Logical Operator259/1 -> Logical Operator271/1
  Logical Operator270/1 -> Logical Operator271/8
  Logical Operator269/1 -> Logical Operator271/7
  Logical Operator268/1 -> Logical Operator271/6
  Logical Operator267/1 -> Logical Operator271/5
  Logical Operator258/1 -> Logical Operator271/12
  Logical Operator257/1 -> Logical Operator271/11
  Logical Operator256/1 -> Logical Operator271/10
  Logical Operator272/1 -> Logical Operator271/9
  Logical Operator264/1 -> Logical Operator271/16
  Logical Operator263/1 -> Logical Operator271/15
  Logical Operator262/1 -> Logical Operator271/14
  Logical Operator261/1 -> Logical Operator271/13
  Select_01(1b)/1 -> Logical Operator263/1, Logical Operator246/1, Logical Operator229/1, Logical Operator212/1, Logical Operator195/1, Logical Operator178/1, Logical Operator161/1, Logical Operator144/1
  Select_05(1b)/1 -> Logical Operator257/1, Logical Operator240/1, Logical Operator223/1, Logical Operator206/1, Logical Operator189/1, Logical Operator172/1, Logical Operator155/1, Logical Operator138/1
  Logical Operator254/1 -> Bus Creator/7
  Logical Operator220/1 -> Bus Creator/5
  Logical Operator186/1 -> Bus Creator/3
  Logical Operator152/1 -> Bus Creator/1

== system Garbage/NOT_USED_Byte_Subtractor ==
  Data_In_A(1B) : Inport
  Data_In_B(1B) : Inport  Port=2
  1_Bit_Subtractor{,1..7} (8 alike) : Arithmetic_Operations_Lib/Arithmetic_Operations_Lib/Bit_Subtractor
  Bit to Integer Converter : simulink/Logic and Bit Operations/Bit to Integer Converter  nbits=8  bitOrder=MSB first  signedOutputValues=Unsigned  outDtype=uint8  outDtypeSigned=Inherit via internal rule
      settings: LibrarySourceBlock=|
      | commutil2/Bit to Integer
      | Converter
  Constant : Constant  Value=0
  Demux : Demux  Outputs=8
  Demux1 : Demux  Outputs=8
  Integer to Bit Converter : simulink/Logic and Bit Operations/Integer to Bit Converter  nbits=8  signedInputValues=Unsigned  bitOrder=MSB first  outDtype=uint8
      settings: LibrarySourceBlock=|
      | commutil2/Integer to Bit
      | Converter
  Integer to Bit Converter1 : simulink/Logic and Bit Operations/Integer to Bit Converter  nbits=8  signedInputValues=Unsigned  bitOrder=MSB first  outDtype=uint8
      settings: LibrarySourceBlock=|
      | commutil2/Integer to Bit
      | Converter
  Mux : Mux  Inputs=8
  Data_Out(1B) : Outport
  Borrow_Out(1b) : Outport  Port=2
  wires:
  Bit to Integer Converter/1 -> Data_Out(1B)/1
  Mux/1 -> Bit to Integer Converter/1
  Integer to Bit Converter1/1 -> Demux1/1
  Data_In_B(1B)/1 -> Integer to Bit Converter1/1
  Integer to Bit Converter/1 -> Demux/1
  Data_In_A(1B)/1 -> Integer to Bit Converter/1
  1_Bit_Subtractor6/2 -> 1_Bit_Subtractor7/3
  1_Bit_Subtractor5/2 -> 1_Bit_Subtractor6/3
  1_Bit_Subtractor4/2 -> 1_Bit_Subtractor5/3
  1_Bit_Subtractor3/2 -> 1_Bit_Subtractor4/3
  1_Bit_Subtractor2/2 -> 1_Bit_Subtractor3/3
  1_Bit_Subtractor1/2 -> 1_Bit_Subtractor2/3
  1_Bit_Subtractor/2 -> 1_Bit_Subtractor1/3
  1_Bit_Subtractor7/2 -> Borrow_Out(1b)/1
  Constant/1 -> 1_Bit_Subtractor/3
  Demux1/8 -> 1_Bit_Subtractor/2
  Demux1/7 -> 1_Bit_Subtractor1/2
  Demux1/6 -> 1_Bit_Subtractor2/2
  Demux1/5 -> 1_Bit_Subtractor3/2
  Demux1/4 -> 1_Bit_Subtractor4/2
  Demux1/3 -> 1_Bit_Subtractor5/2
  Demux1/2 -> 1_Bit_Subtractor6/2
  Demux1/1 -> 1_Bit_Subtractor7/2
  Demux/8 -> 1_Bit_Subtractor/1
  Demux/7 -> 1_Bit_Subtractor1/1
  Demux/6 -> 1_Bit_Subtractor2/1
  Demux/5 -> 1_Bit_Subtractor3/1
  Demux/4 -> 1_Bit_Subtractor4/1
  Demux/3 -> 1_Bit_Subtractor5/1
  Demux/2 -> 1_Bit_Subtractor6/1
  Demux/1 -> 1_Bit_Subtractor7/1
  1_Bit_Subtractor3/1 -> Mux/5
  1_Bit_Subtractor4/1 -> Mux/4
  1_Bit_Subtractor5/1 -> Mux/3
  1_Bit_Subtractor2/1 -> Mux/6
  1_Bit_Subtractor1/1 -> Mux/7
  1_Bit_Subtractor/1 -> Mux/8
  1_Bit_Subtractor6/1 -> Mux/2
  1_Bit_Subtractor7/1 -> Mux/1

== system Garbage/NOT_USED_Counter_2bit ==
  note: 2-bit counter (0..2) with reset
      |
      | Present
      | state
      | Rst=0
      | Rst=1
      | Next state
      |
      | Rst=0
      | Rst=1
      |
      | Q1
      | Q0
      | J1
      | K1
      | J0
      | K0
      | J1
      | K1
      | J0
      | K0
      | Q1
      | Q0
      | Q1
      | Q0
      |
      | 0
      | 0
      | 0
      | X
      | 1
      | X
      | 0
      | X
      | 0
      | X
      | 0
      | 1
      | 0
      | 0
      |
      | 0
      | 1
      | 1
      | X
      | X
      | 1
      | 0
      | X
      | X
      | 1
      | 1
      | 0
      | 0
      | 0
      |
      | 1
      | 0
      | X
      | 1
      | 0
      | X
      | X
      | 1
      | 0
      | X
      | 0
      | 0
      | 0
      | 0
      |
      | 1
      | 1
      | X
      | 1
      | X
      | 1
      | X
      | 1
      | X
      | 1
      | 0
      | 0
      | 0
      | 0
      |
      | J1
      | Q1Q0
      |
      | 00
      | 01
      | 11
      | 10
      |
      | Rst
      | 0
      | 0
      | 1
      | X
      | X
      |
      | 1
      | 0
      | 0
      | X
      | X
      |
      | J1 = ~Rst.Q0
      | K1 = 1
      | J0
      | Q1Q0
      |
      | 00
      | 01
      | 11
      | 10
      |
      | Rst
      | 0
      | 1
      | X
      | X
      | 0
      |
      | 1
      | 0
      | X
      | X
      | 0
      |
      | J0 = ~Rst.~Q1
      | K0 = 1
  Reset : Inport
  CLK : Inport  Port=2
  Bit_0 : simulink_extras/Flip Flops/J-K Flip-Flop  initial_condition=0
  Bit_1 : simulink_extras/Flip Flops/J-K Flip-Flop  initial_condition=0
  Constant2 : Constant  OutDataTypeStr=boolean
  Constant3 : Constant  OutDataTypeStr=boolean
  Logical Operator : Logic  Operator=NOT  IconShape=distinctive  AllPortsSameDT=off  OutDataTypeStr=boolean
  Logical Operator1 : Logic  IconShape=distinctive  AllPortsSameDT=off  OutDataTypeStr=boolean
  Logical Operator2 : Logic  IconShape=distinctive  AllPortsSameDT=off  OutDataTypeStr=boolean
  Terminator1 : Terminator
  Bit1 : Outport
  Bit0 : Outport  Port=2
  wires:
  Constant2/1 -> Bit_1/3
  Constant3/1 -> Bit_0/3
  Bit_0/1 -> Logical Operator1/1, Bit0/1
  Bit_1/2 -> Logical Operator2/2
  CLK/1 -> Bit_0/2, Bit_1/2
  Bit_1/1 -> Bit1/1
  Bit_0/2 -> Terminator1/1
  Logical Operator1/1 -> Bit_1/1
  Reset/1 -> Logical Operator/1
  Logical Operator/1 -> Logical Operator1/2, Logical Operator2/1
  Logical Operator2/1 -> Bit_0/1

== system Garbage/NOT_USED_Counter_3bit ==
  note: J1
      | Q2/Q1
      |
      | 00
      | 01
      | 11
      | 10
      |
      | Rst/Q0
      | 00
      | 0
      | X
      | X
      | 0
      |
      | 01
      | 1
      | X
      | X
      | 0
      |
      | 11
      | 0
      | X
      | X
      | 0
      |
      | 10
      | 0
      | X
      | X
      | 0
      |
      | J1 = ~Rst.~Q2.Q0
  note: J2
      | Q2/Q1
      |
      | 00
      | 01
      | 11
      | 10
      |
      | Rst/Q0
      | 00
      | 0
      | 0
      | X
      | X
      |
      | 01
      | 0
      | 1
      | X
      | X
      |
      | 11
      | 0
      | 0
      | X
      | X
      |
      | 10
      | 0
      | 0
      | X
      | X
      |
      | J2 = ~Rst.Q1.Q0
  note: Present state
      | Rst=0
      | Rst=1
      | Next State
      |
      | Rst=0
      | Rst=1
      |
      | Q2
      | Q1
      | Q0
      | J2
      | K2
      | J1
      | K1
      | J0
      | K0
      | J2
      | K2
      | J1
      | K1
      | J0
      | K0
      | Q2
      | Q1
      | Q0
      | Q2
      | Q1
      | Q0
      |
      | 0
      | 0
      | 0
      | 0
      | X
      | 0
      | X
      | 1
      | X
      | 0
      | X
      | 0
      | X
      | 0
      | X
      | 0
      | 0
      | 1
      | 0
      | 0
      | 0
      |
      | 0
      | 0
      | 1
      | 0
      | X
      | 1
      | X
      | X
      | 1
      | 0
      | X
      | 0
      | X
      | X
      | 1
      | 0
      | 1
      | 0
      | 0
      | 0
      | 0
      |
      | 0
      | 1
      | 0
      | 0
      | X
      | X
      | 0
      | 1
      | X
      | 0
      | X
      | X
      | 1
      | 0
      | X
      | 0
      | 1
      | 1
      | 0
      | 0
      | 0
      |
      | 0
      | 1
      | 1
      | 1
      | X
      | X
      | 1
      | X
      | 1
      | 0
      | X
      | X
      | 1
      | X
      | 1
      | 1
      | 0
      | 0
      | 0
      | 0
      | 0
      |
      | 1
      | 0
      | 0
      | X
      | 0
      | 0
      | X
      | 1
      | X
      | X
      | 1
      | 0
      | X
      | 0
      | X
      | 1
      | 0
      | 1
      | 0
      | 0
      | 0
      |
      | 1
      | 0
      | 1
      | X
      | 1
      | 0
      | X
      | X
      | 1
      | X
      | 1
      | 0
      | X
      | X
      | 1
      | 0
      | 0
      | 0
      | 0
      | 0
      | 0
      |
      | 1
      | 1
      | 0
      | X
      | 1
      | X
      | 1
      | 0
      | X
      | X
      | 1
      | X
      | 1
      | 0
      | X
      | 0
      | 0
      | 0
      | 0
      | 0
      | 0
      |
      | 1
      | 1
      | 1
      | X
      | 1
      | X
      | 1
      | X
      | 1
      | X
      | 1
      | X
      | 1
      | X
      | 1
      | 0
      | 0
      | 0
      | 0
      | 0
      | 0
  note: 3-bit counter with reset
      | (0 -> 1 -> 2 -> 3 -> 4 -> 5 -> 0)
  note: K2
      | Q2/Q1
      |
      | 00
      | 01
      | 11
      | 10
      |
      | Rst/Q0
      | 00
      | X
      | X
      | 1
      | 0
      |
      | 01
      | X
      | X
      | 1
      | 1
      |
      | 11
      | X
      | X
      | 1
      | 1
      |
      | 10
      | X
      | X
      | 1
      | 1
      |
      | K2 = Rst + Q0 + ~Q2 + Q1
  note: K1
      | Q2/Q1
      |
      | 00
      | 01
      | 11
      | 10
      |
      | Rst/Q0
      | 00
      | X
      | 0
      | 1
      | X
      |
      | 01
      | X
      | 1
      | 1
      | X
      |
      | 11
      | X
      | 1
      | 1
      | X
      |
      | 10
      | X
      | 1
      | 1
      | X
      |
      | K1 = Rst + Q0 + Q2 + ~Q1
  note: J0
      | Q2/Q1
      |
      | 00
      | 01
      | 11
      | 10
      |
      | Rst/Q0
      | 00
      | 1
      | 1
      | 0
      | 1
      |
      | 01
      | X
      | X
      | X
      | X
      |
      | 11
      | X
      | X
      | X
      | X
      |
      | 10
      | 0
      | 0
      | 0
      | 0
      |
      | J0 = (~Q2 + ~Q1).(~Rst + Q0)
      | K0 = 1
  Reset : Inport
  CLK : Inport  Port=2
  Bit_0 : simulink_extras/Flip Flops/J-K Flip-Flop  initial_condition=0
  Bit_1 : simulink_extras/Flip Flops/J-K Flip-Flop  initial_condition=0
  Bit_2 : simulink_extras/Flip Flops/J-K Flip-Flop  initial_condition=0
  Constant3 : Constant  OutDataTypeStr=boolean
  Logical Operator : Logic  Operator=NOT  IconShape=distinctive  AllPortsSameDT=off  OutDataTypeStr=boolean
  Logical Operator1 : Logic  Inputs=3  IconShape=distinctive  AllPortsSameDT=off  OutDataTypeStr=boolean
  Logical Operator2 : Logic  IconShape=distinctive  AllPortsSameDT=off  OutDataTypeStr=boolean
  Logical Operator3 : Logic  Operator=OR  IconShape=distinctive  AllPortsSameDT=off  OutDataTypeStr=boolean
  Logical Operator5 : Logic  Operator=OR  Inputs=4  IconShape=distinctive  AllPortsSameDT=off  OutDataTypeStr=boolean
  Logical Operator6 : Logic  Inputs=3  IconShape=distinctive  AllPortsSameDT=off  OutDataTypeStr=boolean
  Logical Operator7 : Logic  Operator=OR  Inputs=4  IconShape=distinctive  AllPortsSameDT=off  OutDataTypeStr=boolean
  Logical Operator8 : Logic  Operator=OR  IconShape=distinctive  AllPortsSameDT=off  OutDataTypeStr=boolean
  Terminator1 : Terminator
  Bit2 : Outport
  Bit1 : Outport  Port=2
  Bit0 : Outport  Port=3
  wires:
  CLK/1 -> Bit_2/2, Bit_1/2, Bit_0/2
  Constant3/1 -> Bit_0/3
  Reset/1 -> Logical Operator/1, Logical Operator5/3, Logical Operator7/3
  Logical Operator/1 -> Logical Operator8/2, Logical Operator1/2, Logical Operator6/2
  Bit_2/1 -> Bit2/1, Logical Operator5/2
  Logical Operator1/1 -> Bit_1/1
  Bit_2/2 -> Logical Operator3/2, Logical Operator7/4, Logical Operator1/3
  Bit_0/1 -> Logical Operator8/1, Logical Operator6/1, Logical Operator7/1, Logical Operator1/1, Logical Operator5/1, Bit0/1
  Logical Operator5/1 -> Bit_1/3
  Bit_1/2 -> Logical Operator3/1, Logical Operator5/4
  Logical Operator6/1 -> Bit_2/1
  Logical Operator7/1 -> Bit_2/3
  Bit_1/1 -> Bit1/1, Logical Operator7/2, Logical Operator6/3
  Logical Operator8/1 -> Logical Operator2/1
  Logical Operator3/1 -> Logical Operator2/2
  Logical Operator2/1 -> Bit_0/1
  Bit_0/2 -> Terminator1/1

== system Garbage/NOT_USED_Counter_4bit ==
  note: K3 = Rst + Q0 + ~Q3 + Q2 + Q1
      | J3 = ~Rst.Q0.~Q3.Q2.Q1
      |
      | J2 = ~Rst.Q0.~Q3.~Q2.Q1
      | K2 = ~Rst.Q0.~Q3.Q2.Q1 + Rst + Q3
      |
      | J1 = ~Rst.Q0.~Q3
      | K1 = Rst + Q0 + Q3
      |
      | J0 = ~Rst.~Q0.~Q3 + ~Rst.~Q0.Q3.~Q2.~Q1 =
      | = ~Rst.~Q0.(~Q3 + Q3.~Q2.~Q1)
      | K0 = 1
  note: Present state
      | Rst=0
      | Rst=1
      | Next state
      |
      | Rst=0
      | Rst=1
      |
      | Q3
      | Q2
      | Q1
      | Q0
      | J3
      | K3
      | J2
      | K2
      | J1
      | K1
      | J0
      | K0
      | J3
      | K3
      | J2
      | K2
      | J1
      | K1
      | J0
      | K0
      | Q3
      | Q2
      | Q1
      | Q0
      | Q3
      | Q2
      | Q1
      | Q0
      |
      | 0
      | 0
      | 0
      | 0
      | 0
      | X
      | 0
      | X
      | 0
      | X
      | 1
      | X
      | 0
      | X
      | 0
      | X
      | 0
      | X
      | 0
      | X
      | 0
      | 0
      | 0
      | 1
      | 0
      | 0
      | 0
      | 0
      |
      | 0
      | 0
      | 0
      | 1
      | 0
      | X
      | 0
      | X
      | 1
      | X
      | X
      | 1
      | 0
      | X
      | 0
      | X
      | 0
      | X
      | X
      | 1
      | 0
      | 0
      | 1
      | 0
      | 0
      | 0
      | 0
      | 0
      |
      | 0
      | 0
      | 1
      | 0
      | 0
      | X
      | 0
      | X
      | X
      | 0
      | 1
      | X
      | 0
      | X
      | 0
      | X
      | X
      | 1
      | 0
      | X
      | 0
      | 0
      | 1
      | 1
      | 0
      | 0
      | 0
      | 0
      |
      | 0
      | 0
      | 1
      | 1
      | 0
      | X
      | 1
      | X
      | X
      | 1
      | X
      | 1
      | 0
      | X
      | 0
      | X
      | X
      | 1
      | X
      | 1
      | 0
      | 1
      | 0
      | 0
      | 0
      | 0
      | 0
      | 0
      |
      | 0
      | 1
      | 0
      | 0
      | 0
      | X
      | X
      | 0
      | 0
      | X
      | 1
      | X
      | 0
      | X
      | X
      | 1
      | 0
      | X
      | 0
      | X
      | 0
      | 1
      | 0
      | 1
      | 0
      | 0
      | 0
      | 0
      |
      | 0
      | 1
      | 0
      | 1
      | 0
      | X
      | X
      | 0
      | 1
      | X
      | X
      | 1
      | 0
      | X
      | X
      | 1
      | 0
      | X
      | X
      | 1
      | 0
      | 1
      | 1
      | 0
      | 0
      | 0
      | 0
      | 0
      |
      | 0
      | 1
      | 1
      | 0
      | 0
      | X
      | X
      | 0
      | X
      | 0
      | 1
      | X
      | 0
      | X
      | X
      | 1
      | X
      | 1
      | 0
      | X
      | 0
      | 1
      | 1
      | 1
      | 0
      | 0
      | 0
      | 0
      |
      | 0
      | 1
      | 1
      | 1
      | 1
      | X
      | X
      | 1
      | X
      | 1
      | X
      | 1
      | 0
      | X
      | X
      | 1
      | X
      | 1
      | X
      | 1
      | 1
      | 0
      | 0
      | 0
      | 0
      | 0
      | 0
      | 0
      |
      | 1
      | 0
      | 0
      | 0
      | X
      | 0
      | 0
      | X
      | 0
      | X
      | 1
      | X
      | X
      | 1
      | 0
      | X
      | 0
      | X
      | 0
      | X
      | 1
      | 0
      | 0
      | 1
      | 0
      | 0
      | 0
      | 0
      |
      | 1
      | 0
      | 0
      | 1
      | X
      | 1
      | 0
      | X
      | 0
      | X
      | X
      | 1
      | X
      | 1
      | 0
      | X
      | 0
      | X
      | X
      | 1
      | 0
      | 0
      | 0
      | 0
      | 0
      | 0
      | 0
      | 0
      |
      | 1
      | 0
      | 1
      | 0
      | X
      | 1
      | 0
      | X
      | X
      | 1
      | 0
      | X
      | X
      | 1
      | 0
      | X
      | X
      | 1
      | 0
      | X
      | 0
      | 0
      | 0
      | 0
      | 0
      | 0
      | 0
      | 0
      |
      | 1
      | 0
      | 1
      | 1
      | X
      | 1
      | 0
      | X
      | X
      | 1
      | X
      | 1
      | X
      | 1
      | 0
      | X
      | X
      | 1
      | X
      | 1
      | 0
      | 0
      | 0
      | 0
      | 0
      | 0
      | 0
      | 0
      |
      | 1
      | 1
      | 0
      | 0
      | X
      | 1
      | X
      | 1
      | 0
      | X
      | 0
      | X
      | X
      | 1
      | X
      | 1
      | 0
      | X
      | 0
      | X
      | 0
      | 0
      | 0
      | 0
      | 0
      | 0
      | 0
      | 0
      |
      | 1
      | 1
      | 0
      | 1
      | X
      | 1
      | X
      | 1
      | 0
      | X
      | X
      | 1
      | X
      | 1
      | X
      | 1
      | 0
      | X
      | X
      | 1
      | 0
      | 0
      | 0
      | 0
      | 0
      | 0
      | 0
      | 0
      |
      | 1
      | 1
      | 1
      | 0
      | X
      | 1
      | X
      | 1
      | X
      | 1
      | 0
      | X
      | X
      | 1
      | X
      | 1
      | X
      | 1
      | 0
      | X
      | 0
      | 0
      | 0
      | 0
      | 0
      | 0
      | 0
      | 0
      |
      | 1
      | 1
      | 1
      | 1
      | X
      | 1
      | X
      | 1
      | X
      | 1
      | X
      | 1
      | X
      | 1
      | X
      | 1
      | X
      | 1
      | X
      | 1
      | 0
      | 0
      | 0
      | 0
      | 0
      | 0
      | 0
      | 0
  note: 4-bit counter with reset
      | (0 -> 1 -> 2 -> 3 -> 4 -> 5 -> 6 -> 7 -> 8 -> 9 -> 0)
  note: K3
      | Q3/Q2/Q1
      |
      | 000
      | 001
      | 011
      | 010
      | 110
      | 111
      | 101
      | 100
      |
      | Rst/Q0
      | 00
      | x
      | x
      | x
      | x
      | 1
      | 1
      | 1
      | 0
      |
      | 01
      | x
      | x
      | x
      | x
      | 1
      | 1
      | 1
      | 1
      |
      | 11
      | x
      | x
      | x
      | x
      | 1
      | 1
      | 1
      | 1
      |
      | 10
      | x
      | x
      | x
      | x
      | 1
      | 1
      | 1
      | 1
      |
      | K3 = Rst + Q0 + ~Q3 + Q2 + Q1
  note: J2
      | Q3/Q2/Q1
      |
      | 000
      | 001
      | 011
      | 010
      | 110
      | 111
      | 101
      | 100
      |
      | Rst/Q0
      | 00
      | 0
      | 0
      | x
      | x
      | x
      | x
      | 0
      | 0
      |
      | 01
      | 0
      | 1
      | x
      | x
      | x
      | x
      | 0
      | 0
      |
      | 11
      | 0
      | 0
      | x
      | x
      | x
      | x
      | 0
      | 0
      |
      | 10
      | 0
      | 0
      | x
      | x
      | x
      | x
      | 0
      | 0
      |
      | J2 = ~Rst.Q0.~Q3.~Q2.Q1
  note: K2
      | Q3/Q2/Q1
      |
      | 000
      | 001
      | 011
      | 010
      | 110
      | 111
      | 101
      | 100
      |
      | Rst/Q0
      | 00
      | x
      | x
      | 0
      | 0
      | 1
      | 1
      | x
      | x
      |
      | 01
      | x
      | x
      | 1
      | 0
      | 1
      | 1
      | x
      | x
      |
      | 11
      | x
      | x
      | 1
      | 1
      | 1
      | 1
      | x
      | x
      |
      | 10
      | x
      | x
      | 1
      | 1
      | 1
      | 1
      | x
      | x
      |
      | K2 = ~Rst.Q0.~Q3.Q2.Q1 + Rst + Q3
  note: J1
      | Q3/Q2/Q1
      |
      | 000
      | 001
      | 011
      | 010
      | 110
      | 111
      | 101
      | 100
      |
      | Rst/Q0
      | 00
      | 0
      | x
      | x
      | 0
      | 0
      | x
      | x
      | 0
      |
      | 01
      | 1
      | x
      | x
      | 1
      | 0
      | x
      | x
      | 0
      |
      | 11
      | 0
      | x
      | x
      | 0
      | 0
      | x
      | x
      | 0
      |
      | 10
      | 0
      | x
      | x
      | 0
      | 0
      | x
      | x
      | 0
      |
      | J1 = ~Rst.Q0.~Q3
  note: K1
      | Q3/Q2/Q1
      |
      | 000
      | 001
      | 011
      | 010
      | 110
      | 111
      | 101
      | 100
      |
      | Rst/Q0
      | 00
      | x
      | 0
      | 0
      | x
      | x
      | 1
      | 1
      | x
      |
      | 01
      | x
      | 1
      | 1
      | x
      | x
      | 1
      | 1
      | x
      |
      | 11
      | x
      | 1
      | 1
      | x
      | x
      | 1
      | 1
      | x
      |
      | 10
      | x
      | 1
      | 1
      | x
      | x
      | 1
      | 1
      | x
      |
      | K1 = Rst + Q0 + Q3
  note: J0
      | Q3/Q2/Q1
      |
      | 000
      | 001
      | 011
      | 010
      | 110
      | 111
      | 101
      | 100
      |
      | Rst/Q0
      | 00
      | 1
      | 1
      | 1
      | 1
      | 0
      | 0
      | 0
      | 1
      |
      | 01
      | x
      | x
      | x
      | x
      | x
      | x
      | x
      | x
      |
      | 11
      | x
      | x
      | x
      | x
      | x
      | x
      | x
      | x
      |
      | 10
      | 0
      | 0
      | 0
      | 0
      | 0
      | 0
      | 0
      | 0
      |
      | J0 = ~Rst.~Q0.~Q3 + ~Rst.~Q0.Q3.~Q2.~Q1 =
      | = ~Rst.~Q0.(~Q3 + Q3.~Q2.~Q1)
      | K0 = 1
  note: J3
      | Q3/Q2/Q1
      |
      | 000
      | 001
      | 011
      | 010
      | 110
      | 111
      | 101
      | 100
      |
      | Rst/Q0
      | 00
      | 0
      | 0
      | 0
      | 0
      | x
      | x
      | x
      | x
      |
      | 01
      | 0
      | 0
      | 1
      | 0
      | x
      | x
      | x
      | x
      |
      | 11
      | 0
      | 0
      | 0
      | 0
      | x
      | x
      | x
      | x
      |
      | 10
      | 0
      | 0
      | 0
      | 0
      | x
      | x
      | x
      | x
      |
      | J3 = ~Rst.Q0.~Q3.Q2.Q1
  Reset : Inport
  CLK : Inport  Port=2
  Bit_{0..3} (4 alike) : simulink_extras/Flip Flops/J-K Flip-Flop  initial_condition=0
  Constant3 : Constant  OutDataTypeStr=boolean
  Logical Operator : Logic  Operator=NOT  IconShape=distinctive  AllPortsSameDT=off  OutDataTypeStr=boolean
  Logical Operator1 : Logic  Inputs=3  IconShape=distinctive  AllPortsSameDT=off  OutDataTypeStr=boolean
  Logical Operator10 : Logic  Inputs=5  IconShape=distinctive  AllPortsSameDT=off  OutDataTypeStr=boolean
  Logical Operator2 : Logic  Inputs=3  IconShape=distinctive  AllPortsSameDT=off  OutDataTypeStr=boolean
  Logical Operator3 : Logic  Operator=OR  Inputs=5  IconShape=distinctive  AllPortsSameDT=off  OutDataTypeStr=boolean
  Logical Operator4 : Logic  Operator=OR  IconShape=distinctive  AllPortsSameDT=off  OutDataTypeStr=boolean
  Logical Operator5 : Logic  Inputs=5  IconShape=distinctive  AllPortsSameDT=off  OutDataTypeStr=boolean
  Logical Operator6 : Logic  Inputs=5  IconShape=distinctive  AllPortsSameDT=off  OutDataTypeStr=boolean
  Logical Operator7 : Logic  Operator=OR  Inputs=3  IconShape=distinctive  AllPortsSameDT=off  OutDataTypeStr=boolean
  Logical Operator8 : Logic  Operator=OR  Inputs=3  IconShape=distinctive  AllPortsSameDT=off  OutDataTypeStr=boolean
  Logical Operator9 : Logic  Inputs=3  IconShape=distinctive  AllPortsSameDT=off  OutDataTypeStr=boolean
  Bit3 : Outport
  Bit2 : Outport  Port=2
  Bit1 : Outport  Port=3
  Bit0 : Outport  Port=4
  wires:
  CLK/1 -> Bit_3/2, Bit_2/2, Bit_1/2, Bit_0/2
  Constant3/1 -> Bit_0/3
  Logical Operator10/1 -> Bit_3/1
  Logical Operator3/1 -> Bit_3/3
  Bit_3/2 -> Logical Operator5/4, Logical Operator1/3, Logical Operator4/2, Logical Operator6/5, Logical Operator3/5, Logical Operator10/5
  Bit_2/1 -> Bit2/1, Logical Operator6/2, Logical Operator3/1, Logical Operator10/4
  Bit_0/1 -> Bit0/1, Logical Operator1/1, Logical Operator7/1, Logical Operator5/1, Logical Operator6/4, Logical Operator10/2, Logical Operator3/3
  Logical Operator/1 -> Logical Operator10/1, Logical Operator2/1, Logical Operator1/2, Logical Operator5/3, Logical Operator6/1
  Logical Operator5/1 -> Bit_2/1
  Bit_2/2 -> Logical Operator5/5, Logical Operator9/3
  Logical Operator8/1 -> Bit_2/3
  Bit_3/1 -> Bit3/1, Logical Operator7/3, Logical Operator9/1, Logical Operator8/2
  Logical Operator6/1 -> Logical Operator8/3
  Bit_1/1 -> Bit1/1, Logical Operator5/2, Logical Operator10/3, Logical Operator3/2, Logical Operator6/3
  Logical Operator1/1 -> Bit_1/1
  Logical Operator7/1 -> Bit_1/3
  Reset/1 -> Logical Operator/1, Logical Operator7/2, Logical Operator8/1, Logical Operator3/4
  Logical Operator4/1 -> Logical Operator2/2
  Logical Operator2/1 -> Bit_0/1
  Bit_0/2 -> Logical Operator2/3
  Logical Operator9/1 -> Logical Operator4/1
  Bit_1/2 -> Logical Operator9/2
